simulink model "CDF_VSVPWM_Grid_Parallel"  (R2024b) (continued)
== system CDF_VSVPWM_Grid_Parallel/VSVPWM1/生成时间调制波/Ⅳ扇区的调制信号计算/2-2 ==
  time : Inport
  Demux : Demux  Outputs=5
  MATLAB Function : MATLAB Function  TreatAsAtomicUnit=on
  MATLAB Function1 : MATLAB Function  TreatAsAtomicUnit=on
  MATLAB Function2 : MATLAB Function  TreatAsAtomicUnit=on
  Mux : Mux  Inputs=6
  Tabc12 : Outport
  wires:
  Demux/5 -> MATLAB Function/5(T5), MATLAB Function1/5(unused1), MATLAB Function2/5(T5)
  Demux/4 -> MATLAB Function/4(unused2), MATLAB Function1/4(unused), MATLAB Function2/4(T4)
  Demux/2 -> MATLAB Function/2(unused), MATLAB Function1/2(T2), MATLAB Function2/2(unused1)
  Demux/1 -> MATLAB Function/1(T1), MATLAB Function1/1(T1), MATLAB Function2/1(unused)
  Demux/3 -> MATLAB Function/3(unused1), MATLAB Function1/3(T3), MATLAB Function2/3(T3)
  time/1 -> Demux/1
  MATLAB Function1/2(tb2) -> Mux/6
  MATLAB Function/1(ta1) -> Mux/3
  MATLAB Function2/1(tc1) -> Mux/1
  MATLAB Function1/1(tb1) -> Mux/5
  Mux/1 -> Tabc12/1
  MATLAB Function/2(ta2) -> Mux/4
  MATLAB Function2/2(tc2) -> Mux/2

== system CDF_VSVPWM_Grid_Parallel/VSVPWM1/生成时间调制波/Ⅳ扇区的调制信号计算/2-3 ==
  time : Inport
  Demux : Demux  Outputs=5
  MATLAB Function : MATLAB Function  TreatAsAtomicUnit=on
  MATLAB Function1 : MATLAB Function  TreatAsAtomicUnit=on
  MATLAB Function2 : MATLAB Function  TreatAsAtomicUnit=on
  Mux : Mux  Inputs=6
  Tabc12 : Outport
  wires:
  time/1 -> Demux/1
  Demux/3 -> MATLAB Function2/3(T3), MATLAB Function1/3(T3), MATLAB Function/3(unused)
  Demux/1 -> MATLAB Function2/1(unused), MATLAB Function1/1(T1), MATLAB Function/1(T1)
  Demux/2 -> MATLAB Function2/2(T2), MATLAB Function1/2(T2), MATLAB Function/2(T2)
  Demux/4 -> MATLAB Function2/4(T4), MATLAB Function1/4(unused), MATLAB Function/4(unused1)
  Demux/5 -> MATLAB Function2/5(T5), MATLAB Function1/5(unused1), MATLAB Function/5(T5)
  MATLAB Function1/2(tb2) -> Mux/6
  MATLAB Function/1(ta1) -> Mux/3
  MATLAB Function2/1(tc1) -> Mux/1
  MATLAB Function1/1(tb1) -> Mux/5
  Mux/1 -> Tabc12/1
  MATLAB Function/2(ta2) -> Mux/4
  MATLAB Function2/2(tc2) -> Mux/2

== system CDF_VSVPWM_Grid_Parallel/VSVPWM1/生成时间调制波/Ⅳ扇区的调制信号计算/2-4 ==
  time : Inport
  Demux : Demux  Outputs=5
  MATLAB Function : MATLAB Function  TreatAsAtomicUnit=on
  MATLAB Function1 : MATLAB Function  TreatAsAtomicUnit=on
  MATLAB Function2 : MATLAB Function  TreatAsAtomicUnit=on
  Mux : Mux  Inputs=6
  Tabc12 : Outport
  wires:
  Demux/5 -> MATLAB Function/5(T5), MATLAB Function1/5(unused), MATLAB Function2/5(T5)
  Demux/4 -> MATLAB Function/4(T4), MATLAB Function1/4(T4), MATLAB Function2/4(T4)
  Demux/2 -> MATLAB Function/2(unused), MATLAB Function1/2(T2), MATLAB Function2/2(unused1)
  Demux/1 -> MATLAB Function/1(T1), MATLAB Function1/1(T1), MATLAB Function2/1(unused)
  Demux/3 -> MATLAB Function/3(unused1), MATLAB Function1/3(T3), MATLAB Function2/3(T3)
  time/1 -> Demux/1
  MATLAB Function1/2(tb2) -> Mux/6
  MATLAB Function/1(ta1) -> Mux/3
  MATLAB Function2/1(tc1) -> Mux/1
  MATLAB Function1/1(tb1) -> Mux/5
  Mux/1 -> Tabc12/1
  MATLAB Function/2(ta2) -> Mux/4
  MATLAB Function2/2(tc2) -> Mux/2

== system CDF_VSVPWM_Grid_Parallel/VSVPWM1/生成时间调制波/Ⅳ扇区的调制信号计算/2-5 ==
  time : Inport
  Demux : Demux  Outputs=5
  MATLAB Function : MATLAB Function  TreatAsAtomicUnit=on
  MATLAB Function1 : MATLAB Function  TreatAsAtomicUnit=on
  MATLAB Function2 : MATLAB Function  TreatAsAtomicUnit=on
  Mux : Mux  Inputs=6
  Tabc12 : Outport
  wires:
  time/1 -> Demux/1
  Demux/3 -> MATLAB Function2/3(T3), MATLAB Function1/3(T3), MATLAB Function/3(unused)
  Demux/1 -> MATLAB Function2/1(unused), MATLAB Function1/1(T1), MATLAB Function/1(T1)
  Demux/2 -> MATLAB Function2/2(T2), MATLAB Function1/2(T2), MATLAB Function/2(T2)
  Demux/4 -> MATLAB Function2/4(T4), MATLAB Function1/4(T4), MATLAB Function/4(T4)
  Demux/5 -> MATLAB Function2/5(T5), MATLAB Function1/5(unused), MATLAB Function/5(T5)
  MATLAB Function1/2(tb2) -> Mux/6
  MATLAB Function/1(ta1) -> Mux/3
  MATLAB Function2/1(tc1) -> Mux/1
  MATLAB Function1/1(tb1) -> Mux/5
  Mux/1 -> Tabc12/1
  MATLAB Function/2(ta2) -> Mux/4
  MATLAB Function2/2(tc2) -> Mux/2

== system CDF_VSVPWM_Grid_Parallel/VSVPWM1/生成时间调制波/Ⅴ扇区的调制信号计算/1-1 ==
  time : Inport
  Demux : Demux  Outputs=5
  MATLAB Function : MATLAB Function  TreatAsAtomicUnit=on
  MATLAB Function1 : MATLAB Function  TreatAsAtomicUnit=on
  MATLAB Function2 : MATLAB Function  TreatAsAtomicUnit=on
  Mux : Mux  Inputs=6
  Scope : Scope
  Tabc12 : Outport
  wires:
  Demux/5 -> MATLAB Function/5(unused2), MATLAB Function1/5(T5), MATLAB Function2/5(T5)
  Demux/4 -> MATLAB Function/4(unused1), MATLAB Function1/4(unused2), MATLAB Function2/4(T4)
  Demux/3 -> MATLAB Function/3(unused), MATLAB Function1/3(unused1), MATLAB Function2/3(unused2)
  Demux/2 -> MATLAB Function/2(T2), MATLAB Function1/2(unused), MATLAB Function2/2(unused1)
  Demux/1 -> MATLAB Function/1(T1), MATLAB Function1/1(T1), MATLAB Function2/1(unused)
  Mux/1 -> Scope/1, Tabc12/1
  time/1 -> Demux/1
  MATLAB Function/1(ta1) -> Mux/5
  MATLAB Function/2(ta2) -> Mux/6
  MATLAB Function1/1(tb1) -> Mux/1
  MATLAB Function1/2(tb2) -> Mux/2
  MATLAB Function2/1(tc1) -> Mux/3
  MATLAB Function2/2(tc2) -> Mux/4

== system CDF_VSVPWM_Grid_Parallel/VSVPWM1/生成时间调制波/Ⅴ扇区的调制信号计算/1-2 ==
  time : Inport
  Demux : Demux  Outputs=5
  MATLAB Function : MATLAB Function  TreatAsAtomicUnit=on
  MATLAB Function1 : MATLAB Function  TreatAsAtomicUnit=on
  MATLAB Function2 : MATLAB Function  TreatAsAtomicUnit=on
  Mux : Mux  Inputs=6
  Tabc12 : Outport
  wires:
  time/1 -> Demux/1
  Demux/3 -> MATLAB Function2/3(T3), MATLAB Function1/3(unused1), MATLAB Function/3(T3)
  Mux/1 -> Tabc12/1
  Demux/1 -> MATLAB Function2/1(unused), MATLAB Function1/1(T1), MATLAB Function/1(T1)
  Demux/2 -> MATLAB Function2/2(unused1), MATLAB Function1/2(unused), MATLAB Function/2(T2)
  Demux/4 -> MATLAB Function2/4(T4), MATLAB Function1/4(unused2), MATLAB Function/4(unused)
  Demux/5 -> MATLAB Function2/5(T5), MATLAB Function1/5(T5), MATLAB Function/5(unused1)
  MATLAB Function/1(ta1) -> Mux/5
  MATLAB Function/2(ta2) -> Mux/6
  MATLAB Function1/1(tb1) -> Mux/1
  MATLAB Function1/2(tb2) -> Mux/2
  MATLAB Function2/1(tc1) -> Mux/3
  MATLAB Function2/2(tc2) -> Mux/4

== system CDF_VSVPWM_Grid_Parallel/VSVPWM1/生成时间调制波/Ⅴ扇区的调制信号计算/1-3 ==
  time : Inport
  Demux : Demux  Outputs=5
  MATLAB Function : MATLAB Function  TreatAsAtomicUnit=on
  MATLAB Function1 : MATLAB Function  TreatAsAtomicUnit=on
  MATLAB Function2 : MATLAB Function  TreatAsAtomicUnit=on
  Mux : Mux  Inputs=6
  Tabc12 : Outport
  wires:
  Demux/5 -> MATLAB Function/5(unused), MATLAB Function1/5(T5), MATLAB Function2/5(T5)
  Demux/4 -> MATLAB Function/4(T4), MATLAB Function1/4(T4), MATLAB Function2/4(T4)
  Demux/2 -> MATLAB Function/2(T2), MATLAB Function1/2(unused), MATLAB Function2/2(unused1)
  Demux/1 -> MATLAB Function/1(T1), MATLAB Function1/1(T1), MATLAB Function2/1(unused)
  Mux/1 -> Tabc12/1
  Demux/3 -> MATLAB Function/3(T3), MATLAB Function1/3(unused1), MATLAB Function2/3(T3)
  time/1 -> Demux/1
  MATLAB Function/1(ta1) -> Mux/5
  MATLAB Function/2(ta2) -> Mux/6
  MATLAB Function1/1(tb1) -> Mux/1
  MATLAB Function1/2(tb2) -> Mux/2
  MATLAB Function2/1(tc1) -> Mux/3
  MATLAB Function2/2(tc2) -> Mux/4

== system CDF_VSVPWM_Grid_Parallel/VSVPWM1/生成时间调制波/Ⅴ扇区的调制信号计算/1-4 ==
  time : Inport
  Demux : Demux  Outputs=5
  MATLAB Function : MATLAB Function  TreatAsAtomicUnit=on
  MATLAB Function1 : MATLAB Function  TreatAsAtomicUnit=on
  MATLAB Function2 : MATLAB Function  TreatAsAtomicUnit=on
  Mux : Mux  Inputs=6
  Tabc12 : Outport
  wires:
  time/1 -> Demux/1
  Demux/3 -> MATLAB Function2/3(T3), MATLAB Function1/3(unused), MATLAB Function/3(T3)
  Mux/1 -> Tabc12/1
  Demux/1 -> MATLAB Function2/1(unused), MATLAB Function1/1(T1), MATLAB Function/1(T1)
  Demux/2 -> MATLAB Function2/2(T2), MATLAB Function1/2(T2), MATLAB Function/2(T2)
  Demux/4 -> MATLAB Function2/4(T4), MATLAB Function1/4(unused1), MATLAB Function/4(unused)
  Demux/5 -> MATLAB Function2/5(T5), MATLAB Function1/5(T5), MATLAB Function/5(unused1)
  MATLAB Function/1(ta1) -> Mux/5
  MATLAB Function/2(ta2) -> Mux/6
  MATLAB Function1/1(tb1) -> Mux/1
  MATLAB Function1/2(tb2) -> Mux/2
  MATLAB Function2/1(tc1) -> Mux/3
  MATLAB Function2/2(tc2) -> Mux/4

== system CDF_VSVPWM_Grid_Parallel/VSVPWM1/生成时间调制波/Ⅴ扇区的调制信号计算/1-5 ==
  time : Inport
  Demux : Demux  Outputs=5
  MATLAB Function : MATLAB Function  TreatAsAtomicUnit=on
  MATLAB Function1 : MATLAB Function  TreatAsAtomicUnit=on
  MATLAB Function2 : MATLAB Function  TreatAsAtomicUnit=on
  Mux : Mux  Inputs=6
  Tabc12 : Outport
  wires:
  Demux/5 -> MATLAB Function/5(unused), MATLAB Function1/5(T5), MATLAB Function2/5(T5)
  Demux/4 -> MATLAB Function/4(T4), MATLAB Function1/4(T4), MATLAB Function2/4(T4)
  Demux/2 -> MATLAB Function/2(T2), MATLAB Function1/2(T2), MATLAB Function2/2(T2)
  Demux/1 -> MATLAB Function/1(T1), MATLAB Function1/1(T1), MATLAB Function2/1(unused)
  Demux/3 -> MATLAB Function/3(T3), MATLAB Function1/3(unused), MATLAB Function2/3(T3)
  time/1 -> Demux/1
  MATLAB Function1/2(tb2) -> Mux/2
  MATLAB Function2/1(tc1) -> Mux/3
  Mux/1 -> Tabc12/1
  MATLAB Function/1(ta1) -> Mux/5
  MATLAB Function2/2(tc2) -> Mux/4
  MATLAB Function/2(ta2) -> Mux/6
  MATLAB Function1/1(tb1) -> Mux/1

== system CDF_VSVPWM_Grid_Parallel/VSVPWM1/生成时间调制波/Ⅵ扇区的调制信号计算/2-1 ==
  time : Inport
  Demux : Demux  Outputs=5
  MATLAB Function : MATLAB Function  TreatAsAtomicUnit=on
  MATLAB Function1 : MATLAB Function  TreatAsAtomicUnit=on
  MATLAB Function2 : MATLAB Function  TreatAsAtomicUnit=on
  Mux : Mux  Inputs=6
  Tabc12 : Outport
  wires:
  time/1 -> Demux/1
  Demux/3 -> MATLAB Function2/3(unused2), MATLAB Function1/3(unused), MATLAB Function/3(unused1)
  Demux/1 -> MATLAB Function2/1(unused), MATLAB Function1/1(T1), MATLAB Function/1(T1)
  Demux/2 -> MATLAB Function2/2(unused1), MATLAB Function1/2(T2), MATLAB Function/2(unused)
  Demux/4 -> MATLAB Function2/4(T4), MATLAB Function1/4(unused1), MATLAB Function/4(unused2)
  Demux/5 -> MATLAB Function2/5(T5), MATLAB Function1/5(unused2), MATLAB Function/5(T5)
  MATLAB Function1/2(tb2) -> Mux/2
  MATLAB Function1/1(tb1) -> Mux/1
  MATLAB Function2/2(tc2) -> Mux/4
  MATLAB Function2/1(tc1) -> Mux/3
  MATLAB Function/2(ta2) -> Mux/6
  MATLAB Function/1(ta1) -> Mux/5
  Mux/1 -> Tabc12/1

== system CDF_VSVPWM_Grid_Parallel/VSVPWM1/生成时间调制波/Ⅵ扇区的调制信号计算/2-2 ==
  time : Inport
  Demux : Demux  Outputs=5
  MATLAB Function : MATLAB Function  TreatAsAtomicUnit=on
  MATLAB Function1 : MATLAB Function  TreatAsAtomicUnit=on
  MATLAB Function2 : MATLAB Function  TreatAsAtomicUnit=on
  Mux : Mux  Inputs=6
  Tabc12 : Outport
  wires:
  Demux/5 -> MATLAB Function/5(T5), MATLAB Function1/5(unused1), MATLAB Function2/5(T5)
  Demux/4 -> MATLAB Function/4(unused2), MATLAB Function1/4(unused), MATLAB Function2/4(T4)
  Demux/2 -> MATLAB Function/2(unused), MATLAB Function1/2(T2), MATLAB Function2/2(unused1)
  Demux/1 -> MATLAB Function/1(T1), MATLAB Function1/1(T1), MATLAB Function2/1(unused)
  Demux/3 -> MATLAB Function/3(unused1), MATLAB Function1/3(T3), MATLAB Function2/3(T3)
  time/1 -> Demux/1
  MATLAB Function1/2(tb2) -> Mux/2
  MATLAB Function1/1(tb1) -> Mux/1
  MATLAB Function2/2(tc2) -> Mux/4
  MATLAB Function2/1(tc1) -> Mux/3
  MATLAB Function/2(ta2) -> Mux/6
  MATLAB Function/1(ta1) -> Mux/5
  Mux/1 -> Tabc12/1

== system CDF_VSVPWM_Grid_Parallel/VSVPWM1/生成时间调制波/Ⅵ扇区的调制信号计算/2-3 ==
  time : Inport
  Demux : Demux  Outputs=5
  MATLAB Function : MATLAB Function  TreatAsAtomicUnit=on
  MATLAB Function1 : MATLAB Function  TreatAsAtomicUnit=on
  MATLAB Function2 : MATLAB Function  TreatAsAtomicUnit=on
  Mux : Mux  Inputs=6
  Tabc12 : Outport
  wires:
  time/1 -> Demux/1
  Demux/3 -> MATLAB Function2/3(T3), MATLAB Function1/3(T3), MATLAB Function/3(unused)
  Demux/1 -> MATLAB Function2/1(unused), MATLAB Function1/1(T1), MATLAB Function/1(T1)
  Demux/2 -> MATLAB Function2/2(T2), MATLAB Function1/2(T2), MATLAB Function/2(T2)
  Demux/4 -> MATLAB Function2/4(T4), MATLAB Function1/4(unused), MATLAB Function/4(unused1)
  Demux/5 -> MATLAB Function2/5(T5), MATLAB Function1/5(unused1), MATLAB Function/5(T5)
  MATLAB Function1/2(tb2) -> Mux/2
  MATLAB Function1/1(tb1) -> Mux/1
  MATLAB Function2/2(tc2) -> Mux/4
  MATLAB Function2/1(tc1) -> Mux/3
  MATLAB Function/2(ta2) -> Mux/6
  MATLAB Function/1(ta1) -> Mux/5
  Mux/1 -> Tabc12/1

== system CDF_VSVPWM_Grid_Parallel/VSVPWM1/生成时间调制波/Ⅵ扇区的调制信号计算/2-4 ==
  time : Inport
  Demux : Demux  Outputs=5
  MATLAB Function : MATLAB Function  TreatAsAtomicUnit=on
  MATLAB Function1 : MATLAB Function  TreatAsAtomicUnit=on
  MATLAB Function2 : MATLAB Function  TreatAsAtomicUnit=on
  Mux : Mux  Inputs=6
  Tabc12 : Outport
  wires:
  Demux/5 -> MATLAB Function/5(T5), MATLAB Function1/5(unused), MATLAB Function2/5(T5)
  Demux/4 -> MATLAB Function/4(T4), MATLAB Function1/4(T4), MATLAB Function2/4(T4)
  Demux/2 -> MATLAB Function/2(unused), MATLAB Function1/2(T2), MATLAB Function2/2(unused1)
  Demux/1 -> MATLAB Function/1(T1), MATLAB Function1/1(T1), MATLAB Function2/1(unused)
  Demux/3 -> MATLAB Function/3(unused1), MATLAB Function1/3(T3), MATLAB Function2/3(T3)
  time/1 -> Demux/1
  MATLAB Function1/2(tb2) -> Mux/2
  MATLAB Function1/1(tb1) -> Mux/1
  MATLAB Function2/2(tc2) -> Mux/4
  MATLAB Function2/1(tc1) -> Mux/3
  MATLAB Function/2(ta2) -> Mux/6
  MATLAB Function/1(ta1) -> Mux/5
  Mux/1 -> Tabc12/1

== system CDF_VSVPWM_Grid_Parallel/VSVPWM1/生成时间调制波/Ⅵ扇区的调制信号计算/2-5 ==
  time : Inport
  Demux : Demux  Outputs=5
  MATLAB Function : MATLAB Function  TreatAsAtomicUnit=on
  MATLAB Function1 : MATLAB Function  TreatAsAtomicUnit=on
  MATLAB Function2 : MATLAB Function  TreatAsAtomicUnit=on
  Mux : Mux  Inputs=6
  Tabc12 : Outport
  wires:
  time/1 -> Demux/1
  Demux/3 -> MATLAB Function2/3(T3), MATLAB Function1/3(T3), MATLAB Function/3(unused)
  Demux/1 -> MATLAB Function2/1(unused), MATLAB Function1/1(T1), MATLAB Function/1(T1)
  Demux/2 -> MATLAB Function2/2(T2), MATLAB Function1/2(T2), MATLAB Function/2(T2)
  Demux/4 -> MATLAB Function2/4(T4), MATLAB Function1/4(T4), MATLAB Function/4(T4)
  Demux/5 -> MATLAB Function2/5(T5), MATLAB Function1/5(unused), MATLAB Function/5(T5)
  MATLAB Function1/2(tb2) -> Mux/2
  MATLAB Function1/1(tb1) -> Mux/1
  MATLAB Function2/2(tc2) -> Mux/4
  MATLAB Function2/1(tc1) -> Mux/3
  MATLAB Function/2(ta2) -> Mux/6
  MATLAB Function/1(ta1) -> Mux/5
  Mux/1 -> Tabc12/1

== matlab function CDF_VSVPWM_Grid_Parallel/VSVPWM/生成时间调制波/Ⅱ扇区的调制信号计算/2-2/MATLAB Function ==
  data: T1 input; ta1 output; unused input; unused1 input; unused2 input; T5 input; ta2 output
function [ta1,ta2] = fcn(T1,~,~,~,T5)

ta1 =2*T1;
ta2 =-T5;

== matlab function CDF_VSVPWM_Grid_Parallel/VSVPWM/生成时间调制波/Ⅱ扇区的调制信号计算/2-2/MATLAB Function1 ==
  data: T1 input; tb1 output; T2 input; T3 input; unused input; unused1 input; tb2 output
function [tb1,tb2] = fcn(T1,T2,T3,~,~)

tb1 =2*(T1+T2+T3);
tb2 =0;

== matlab function CDF_VSVPWM_Grid_Parallel/VSVPWM/生成时间调制波/Ⅱ扇区的调制信号计算/2-2/MATLAB Function2 ==
  data: unused input; tc1 output; unused1 input; T3 input; T4 input; T5 input; tc2 output
function [tc1,tc2] = fcn(~,~,T3,T4,T5)

tc2 =-(2*T3+2*T4+T5);
tc1 =0;

== matlab function CDF_VSVPWM_Grid_Parallel/VSVPWM/生成时间调制波/Ⅱ扇区的调制信号计算/2-3/MATLAB Function ==
  data: T1 input; ta1 output; T2 input; unused input; unused1 input; T5 input; ta2 output
function [ta1,ta2] = fcn(T1,T2,~,~,T5)

ta1 =2*(T1+T2);
ta2 =-T5;

== matlab function CDF_VSVPWM_Grid_Parallel/VSVPWM/生成时间调制波/Ⅱ扇区的调制信号计算/2-3/MATLAB Function1 ==
  data: T1 input; tb1 output; T2 input; T3 input; unused input; unused1 input; tb2 output
function [tb1,tb2] = fcn(T1,T2,T3,~,~)

tb1 =2*(T1+T2+T3);
tb2 =0;

== matlab function CDF_VSVPWM_Grid_Parallel/VSVPWM/生成时间调制波/Ⅱ扇区的调制信号计算/2-3/MATLAB Function2 ==
  data: unused input; tc1 output; T2 input; T3 input; T4 input; T5 input; tc2 output
function [tc1,tc2] = fcn(~,T2,T3,T4,T5)

tc2 =-(2*T2+2*T3+2*T4+T5);
tc1 =0;

== matlab function CDF_VSVPWM_Grid_Parallel/VSVPWM/生成时间调制波/Ⅱ扇区的调制信号计算/2-4/MATLAB Function ==
  data: T1 input; ta1 output; unused input; unused1 input; T4 input; T5 input; ta2 output
function [ta1,ta2] = fcn(T1,~,~,T4,T5)

ta1 =2*T1;
ta2 =-(2*T4+T5);

== matlab function CDF_VSVPWM_Grid_Parallel/VSVPWM/生成时间调制波/Ⅱ扇区的调制信号计算/2-4/MATLAB Function1 ==
  data: T1 input; tb1 output; T2 input; T3 input; T4 input; unused input; tb2 output
function [tb1,tb2] = fcn(T1,T2,T3,T4,~)

tb1 =2*(T1+T2+T3+T4);
tb2 =0;

== matlab function CDF_VSVPWM_Grid_Parallel/VSVPWM/生成时间调制波/Ⅱ扇区的调制信号计算/2-4/MATLAB Function2 ==
  data: unused input; tc1 output; unused1 input; T3 input; T4 input; T5 input; tc2 output
function [tc1,tc2] = fcn(~,~,T3,T4,T5)

tc2 =-(2*T3+2*T4+T5);
tc1 =0;

== matlab function CDF_VSVPWM_Grid_Parallel/VSVPWM/生成时间调制波/Ⅱ扇区的调制信号计算/2-5/MATLAB Function ==
  data: T1 input; ta1 output; T2 input; unused input; T4 input; T5 input; ta2 output
function [ta1,ta2] = fcn(T1,T2,~,T4,T5)

ta1 =2*(T1+T2);
ta2 =-(2*T4+T5);

== matlab function CDF_VSVPWM_Grid_Parallel/MATLAB Function ==
  data: dUdc input; k1_inv1 output; iz input; ix1 input; iy1 input; k2_inv1 output
function [k1_inv1, k2_inv1] = AllocationFactorGen_Inv1(dUdc, iz, ix1, iy1)
% CDF-VSVPWM allocation factor generator.
% The iz input keeps the existing external sign preprocessing.

dU_max = 3.5;
Iz_max = 12.0;
K_np0  = 0.16;
K_z0   = 0.025;
tau    = 1.8;

Enp = abs(dUdc) / max(dU_max, eps);
Ez  = abs(iz)   / max(Iz_max, eps);
rho = 1.0 / (1.0 + exp(-tau * (Ez - Enp)));

K_z_dyn  = K_z0  * (1.0 + rho);
K_np_dyn = K_np0 * (2.0 - rho);

np_term_1 = dUdc * sign(ix1);
np_term_2 = -dUdc * sign(iy1);
z_term    = -iz;

if np_term_1 * z_term < 0
    K_np_1 = K_np_dyn;
    K_z_1  = K_z_dyn;
else
    K_np_1 = K_np0;
    K_z_1  = K_z0;
end

if np_term_2 * z_term < 0
    K_np_2 = K_np_dyn;
    K_z_2  = K_z_dyn;
else
    K_np_2 = K_np0;
    K_z_2  = K_z0;
end

k1_raw = 0.5 + K_np_1 * np_term_1 + K_z_1 * z_term;
k2_raw = 0.5 + K_np_2 * np_term_2 + K_z_2 * z_term;

k1_inv1 = min(1.0, max(0.0, k1_raw));
k2_inv1 = min(1.0, max(0.0, k2_raw));
end

== matlab function CDF_VSVPWM_Grid_Parallel/VSVPWM/生成时间调制波/Ⅱ扇区的调制信号计算/2-5/MATLAB Function1 ==
  data: T1 input; tb1 output; T2 input; T3 input; T4 input; unused input; tb2 output
function [tb1,tb2] = fcn(T1,T2,T3,T4,~)

tb1 =2*(T1+T2+T3+T4);
tb2 =0;

== matlab function CDF_VSVPWM_Grid_Parallel/VSVPWM/生成时间调制波/Ⅱ扇区的调制信号计算/2-5/MATLAB Function2 ==
  data: unused input; tc1 output; T2 input; T3 input; T4 input; T5 input; tc2 output
function [tc1,tc2] = fcn(~,T2,T3,T4,T5)

tc2 =-(2*T2+2*T3+2*T4+T5);
tc1 =0;

== matlab function CDF_VSVPWM_Grid_Parallel/VSVPWM/生成时间调制波/Ⅲ扇区的调制信号计算1/1-1/MATLAB Function ==
  data: T1 input; ta1 output; T2 input; unused input; unused1 input; unused2 input; ta2 output
function [ta1,ta2] = fcn(T1,T2,~,~,~)

ta1 =2*(T1+T2);
ta2 =0;

== matlab function CDF_VSVPWM_Grid_Parallel/VSVPWM/生成时间调制波/Ⅲ扇区的调制信号计算1/1-1/MATLAB Function1 ==
  data: T1 input; tb1 output; unused input; unused1 input; unused2 input; T5 input; tb2 output
function [tb1,tb2] = fcn(T1,~,~,~,T5)

tb1 =2*T1;
tb2 =-T5;

== matlab function CDF_VSVPWM_Grid_Parallel/VSVPWM/生成时间调制波/Ⅲ扇区的调制信号计算1/1-1/MATLAB Function2 ==
  data: unused input; tc1 output; unused1 input; unused2 input; T4 input; T5 input; tc2 output
function [tc1,tc2] = fcn(~,~,~,T4,T5)

tc2 =-(2*T4+T5);
tc1 =0;

== matlab function CDF_VSVPWM_Grid_Parallel/VSVPWM/生成时间调制波/Ⅲ扇区的调制信号计算1/1-2/MATLAB Function ==
  data: T1 input; ta1 output; T2 input; T3 input; unused input; unused1 input; ta2 output
function [ta1,ta2] = fcn(T1,T2,T3,~,~)

ta1 =2*(T1+T2+T3);
ta2 =0;

== matlab function CDF_VSVPWM_Grid_Parallel/VSVPWM/生成时间调制波/Ⅲ扇区的调制信号计算1/1-2/MATLAB Function1 ==
  data: T1 input; tb1 output; unused input; unused1 input; unused2 input; T5 input; tb2 output
function [tb1,tb2] = fcn(T1,~,~,~,T5)

tb1 =2*T1;
tb2 =-T5;

== matlab function CDF_VSVPWM_Grid_Parallel/VSVPWM/生成时间调制波/Ⅲ扇区的调制信号计算1/1-2/MATLAB Function2 ==
  data: unused input; tc1 output; unused1 input; T3 input; T4 input; T5 input; tc2 output
function [tc1,tc2] = fcn(~,~,T3,T4,T5)

tc2 =-(2*T3+2*T4+T5);
tc1 =0;

== matlab function CDF_VSVPWM_Grid_Parallel/VSVPWM/生成时间调制波/Ⅲ扇区的调制信号计算1/1-3/MATLAB Function ==
  data: T1 input; ta1 output; T2 input; T3 input; T4 input; unused input; ta2 output
function [ta1,ta2] = fcn(T1,T2,T3,T4,~)

ta1 =2*(T1+T2+T3+T4);
ta2 =0;

== matlab function CDF_VSVPWM_Grid_Parallel/VSVPWM1/大扇区判断/MATLAB Function ==
  data: u input; y output
function y = fcn(u)

y = u*3/pi;

== matlab function CDF_VSVPWM_Grid_Parallel/VSVPWM/生成时间调制波/Ⅲ扇区的调制信号计算1/1-3/MATLAB Function1 ==
  data: T1 input; tb1 output; unused input; unused1 input; T4 input; T5 input; tb2 output
function [tb1,tb2] = fcn(T1,~,~,T4,T5)

tb1 =2*T1;
tb2 =-1*(2*T4+T5);

== matlab function CDF_VSVPWM_Grid_Parallel/VSVPWM/生成时间调制波/Ⅲ扇区的调制信号计算1/1-3/MATLAB Function2 ==
  data: unused input; tc1 output; unused1 input; T3 input; T4 input; T5 input; tc2 output
function [tc1,tc2] = fcn(~,~,T3,T4,T5)

tc2 =-1*(2*T3+2*T4+T5);
tc1 =0;

== matlab function CDF_VSVPWM_Grid_Parallel/VSVPWM1/生成时间调制波/Ⅳ扇区的调制信号计算/2-1/MATLAB Function ==
  data: T1 input; ta1 output; unused input; unused1 input; unused2 input; T5 input; ta2 output
function [ta1,ta2] = fcn(T1,~,~,~,T5)

ta1 =2*T1;
ta2 =-T5;

== matlab function CDF_VSVPWM_Grid_Parallel/VSVPWM1/生成时间调制波/Ⅳ扇区的调制信号计算/2-1/MATLAB Function1 ==
  data: T1 input; tb1 output; T2 input; unused input; unused1 input; unused2 input; tb2 output
function [tb1,tb2] = fcn(T1,T2,~,~,~)

tb1 =2*(T1+T2);
tb2 =0;

== matlab function CDF_VSVPWM_Grid_Parallel/VSVPWM1/生成时间调制波/Ⅳ扇区的调制信号计算/2-1/MATLAB Function2 ==
  data: unused input; tc1 output; unused1 input; unused2 input; T4 input; T5 input; tc2 output
function [tc1,tc2] = fcn(~,~,~,T4,T5)

tc2 =-(2*T4+T5);
tc1 =0;

== matlab function CDF_VSVPWM_Grid_Parallel/VSVPWM1/生成时间调制波/Ⅳ扇区的调制信号计算/2-2/MATLAB Function ==
  data: T1 input; ta1 output; unused input; unused1 input; unused2 input; T5 input; ta2 output
function [ta1,ta2] = fcn(T1,~,~,~,T5)

ta1 =2*T1;
ta2 =-T5;

== matlab function CDF_VSVPWM_Grid_Parallel/4-8 ==
  data: ealphak1 input; ebetak1 input; ialpha input; ibeta input; ialpha_k1_ref input; ibeta_k1_ref input; R input; L input; Ts input; ualpha_ref output; ubeta_ref output
function [ualpha_ref, ubeta_ref] = fcn(ealphak1, ebetak1, ialpha, ibeta, ialpha_k1_ref, ibeta_k1_ref, R, L, Ts)
    % 离散化系数
    K = L/Ts;
    
    % 计算逆变器输出电压参考值 (假设电流正方向为：逆变器 -> 电网)
    ualpha_ref = ealphak1 + ialpha*R + K*(ialpha_k1_ref - ialpha);
    ubeta_ref  = ebetak1  + ibeta*R  + K*(ibeta_k1_ref  - ibeta);
end

== matlab function CDF_VSVPWM_Grid_Parallel/VSVPWM1/生成时间调制波/Ⅳ扇区的调制信号计算/2-2/MATLAB Function1 ==
  data: T1 input; tb1 output; T2 input; T3 input; unused input; unused1 input; tb2 output
function [tb1,tb2] = fcn(T1,T2,T3,~,~)

tb1 =2*(T1+T2+T3);
tb2 =0;

== matlab function CDF_VSVPWM_Grid_Parallel/VSVPWM1/生成时间调制波/Ⅳ扇区的调制信号计算/2-2/MATLAB Function2 ==
  data: unused input; tc1 output; unused1 input; T3 input; T4 input; T5 input; tc2 output
function [tc1,tc2] = fcn(~,~,T3,T4,T5)

tc2 =-(2*T3+2*T4+T5);
tc1 =0;

== matlab function CDF_VSVPWM_Grid_Parallel/VSVPWM1/生成时间调制波/Ⅳ扇区的调制信号计算/2-3/MATLAB Function ==
  data: T1 input; ta1 output; T2 input; unused input; unused1 input; T5 input; ta2 output
function [ta1,ta2] = fcn(T1,T2,~,~,T5)

ta1 =2*(T1+T2);
ta2 =-T5;

== matlab function CDF_VSVPWM_Grid_Parallel/VSVPWM1/生成时间调制波/Ⅳ扇区的调制信号计算/2-3/MATLAB Function1 ==
  data: T1 input; tb1 output; T2 input; T3 input; unused input; unused1 input; tb2 output
function [tb1,tb2] = fcn(T1,T2,T3,~,~)

tb1 =2*(T1+T2+T3);
tb2 =0;

== matlab function CDF_VSVPWM_Grid_Parallel/VSVPWM1/生成时间调制波/Ⅳ扇区的调制信号计算/2-3/MATLAB Function2 ==
  data: unused input; tc1 output; T2 input; T3 input; T4 input; T5 input; tc2 output
function [tc1,tc2] = fcn(~,T2,T3,T4,T5)

tc2 =-(2*T2+2*T3+2*T4+T5);
tc1 =0;

== matlab function CDF_VSVPWM_Grid_Parallel/VSVPWM1/生成时间调制波/Ⅳ扇区的调制信号计算/2-4/MATLAB Function ==
  data: T1 input; ta1 output; unused input; unused1 input; T4 input; T5 input; ta2 output
function [ta1,ta2] = fcn(T1,~,~,T4,T5)

ta1 =2*T1;
ta2 =-(2*T4+T5);

== matlab function CDF_VSVPWM_Grid_Parallel/VSVPWM1/生成时间调制波/Ⅳ扇区的调制信号计算/2-4/MATLAB Function1 ==
  data: T1 input; tb1 output; T2 input; T3 input; T4 input; unused input; tb2 output
function [tb1,tb2] = fcn(T1,T2,T3,T4,~)

tb1 =2*(T1+T2+T3+T4);
tb2 =0;

== matlab function CDF_VSVPWM_Grid_Parallel/VSVPWM1/生成时间调制波/Ⅳ扇区的调制信号计算/2-4/MATLAB Function2 ==
  data: unused input; tc1 output; unused1 input; T3 input; T4 input; T5 input; tc2 output
function [tc1,tc2] = fcn(~,~,T3,T4,T5)

tc2 =-(2*T3+2*T4+T5);
tc1 =0;

== matlab function CDF_VSVPWM_Grid_Parallel/VSVPWM1/生成时间调制波/Ⅳ扇区的调制信号计算/2-5/MATLAB Function ==
  data: T1 input; ta1 output; T2 input; unused input; T4 input; T5 input; ta2 output
function [ta1,ta2] = fcn(T1,T2,~,T4,T5)

ta1 =2*(T1+T2);
ta2 =-(2*T4+T5);

== matlab function CDF_VSVPWM_Grid_Parallel/VSVPWM1/生成时间调制波/Ⅳ扇区的调制信号计算/2-5/MATLAB Function1 ==
  data: T1 input; tb1 output; T2 input; T3 input; T4 input; unused input; tb2 output
function [tb1,tb2] = fcn(T1,T2,T3,T4,~)

tb1 =2*(T1+T2+T3+T4);
tb2 =0;

== matlab function CDF_VSVPWM_Grid_Parallel/VSVPWM1/生成时间调制波/Ⅳ扇区的调制信号计算/2-5/MATLAB Function2 ==
  data: unused input; tc1 output; T2 input; T3 input; T4 input; T5 input; tc2 output
function [tc1,tc2] = fcn(~,T2,T3,T4,T5)

tc2 =-(2*T2+2*T3+2*T4+T5);
tc1 =0;
